simulink model "TosAeb"  (R2021a)
settings: Solver=FixedStepDiscrete  StartTime=0.0  StopTime=inf  FixedStep=0.01  RelTol=1e-3
undefined symbols (not defined in the file or in its folder): ACCModSts, AccSts1, AEB_ObjectMovingOrientation, AEB_ObjectMovingState, CN_BTCActive, CN_CurrentGearR, CN_CurrentGearR_DHT, CN_PTCActive, CN_VDCActive, CN_VSC_EspOff, CN_VSC_FCW_AEB_Error, CN_VSC_FCW_AEB_NoDispay, CN_VSC_FCW_AEB_PerfmDgdtn, CN_VSC_FCW_AEB_TempUnAvlbl, CN_VSC_VehStandstill, CNaccelAvoidMax, CNaccelAvoidMin, CNbrakePedalPress, CNcomfortStrLeftAccelMax, CNcomfortStrLeftAccelMin, CNcomfortStrRightAccelMax, CNcomfortStrRightAccelMin, CNDrvDoorOpen, CNDrvModeEco, CNDrvModeNorm, CNDrvModeOther, CNDrvModeSport, CNDrvSBRreminder, CNNull, CNobjInvalidID, CNPassDoorOpen, CNPassSBRreminder, CNPEBSAccelNegInf, CNPEBSAccelNull, CNPEBSTimeInf, CNPEBSTimeNull, CNSTOPMAX, CNSTOPNULL, CNsubjWidth, CNSwtOFF, CNSwtON, CNTTCMAX, CNTTCNULL, CNVehConfigAEBCycNone, CNVehConfigAEBNone, CNVehConfigAEBPedNone, CNVehConfigFCWNone, CNVehConfigJANone, CollisionSeverity, FALSE, FCWSnvty, JACycObjCalcInfoDefaultParams, JAPedObjCalcInfoDefaultParams, JAVehObjCalcInfoDefaultParams, latCycObjCalcInfoDefaultParams, latPedObjCalcInfoDefaultParams, longObjCalcInfoDefaultParams, ModSts, movingStatus, ObjectType ... (+1112)
libraries: simulink x3031, AEBSFunctionLib x50, Ta_Library x33, AEBSStateMachineLib x23, Mylibrary x12, simulink_extras x7, ObjSelect_lib x3, dspstat3 x2
(part 82 of 102)

== system TosAeb/AEB_Objsel/TA_Processor/AEB_ObjSelect/TargetInfoCheck/Data_precess/TA_EffectCalculate/Obj_FilterFlgCalculate/Long_ObjFilter/TTR_Calculate/CalculateTTRAndTTM_else/LongitudinalTTRComputer/SelectTTI/ObjectOnlyWillStop ==
  StationaryObjectTTI : Inport
  StationaryObjectSolutionExists : Inport  Port=2
  Action Port : ActionPort  ActionPortLabel=elseif(u3~=0)
  NoOperation : Subsystem  mask(noop){}
      mask help: No operation block to to hadle when a signal is passed unchanged between ports with different names
      mask Display: disp('NOOP')
  NoOperation1 : Subsystem  mask(noop){}
      mask help: No operation block to to hadle when a signal is passed unchanged between ports with different names
      mask Display: disp('NOOP')
  TimeToIntersection : Outport
  SolutionExists : Outport  Port=2
  wires:
  NoOperation/1(Out) -> TimeToIntersection/1  "TimeToIntersection"
  StationaryObjectTTI/1 -> NoOperation/1(In)
  NoOperation1/1(Out) -> SolutionExists/1  "SolutionExists"
  StationaryObjectSolutionExists/1 -> NoOperation1/1(In)

== system TosAeb/AEB_Objsel/TA_Processor/AEB_ObjSelect/TargetInfoCheck/Data_precess/TA_EffectCalculate/Obj_FilterFlgCalculate/Long_ObjFilter/TTR_Calculate/CalculateTTRAndTTM_else/Obj_AbsInfo/LongitudinalEdgeToEdgeDistances/Rename ==
  note: Block intended for fooling the model advisor, which want the signal names to
      | match the port names and which only allows signal renaming in combination
      | with transformative operations. The block has no effect on the signal and is
      | intended to replace, e.g., gain blocks with one as its multiplication factor.
      | See MAAB jm_0010: Port block names in Simulink models.
  Name_in : Inport
      settings: AttributesFormatString=DT=%<OutDataTypeStr>
  Name_out : Outport
      settings: AttributesFormatString=DT=%<OutDataTypeStr>
  wires:
  Name_in/1 -> Name_out/1  "Name"

== system TosAeb/AEB_Objsel/TA_Processor/AEB_ObjSelect/TargetInfoCheck/Data_precess/TA_EffectCalculate/Obj_FilterFlgCalculate/Long_ObjFilter/TTR_Calculate/CalculateTTRAndTTM_else/Obj_AbsInfo/LongitudinalEdgeToEdgeDistances/Rename1 ==
  note: Block intended for fooling the model advisor, which want the signal names to
      | match the port names and which only allows signal renaming in combination
      | with transformative operations. The block has no effect on the signal and is
      | intended to replace, e.g., gain blocks with one as its multiplication factor.
      | See MAAB jm_0010: Port block names in Simulink models.
  Name_in : Inport
      settings: AttributesFormatString=DT=%<OutDataTypeStr>
  Name_out : Outport
      settings: AttributesFormatString=DT=%<OutDataTypeStr>
  wires:
  Name_in/1 -> Name_out/1  "Name"

== system TosAeb/AEB_Objsel/TA_Processor/AEB_ObjSelect/TargetInfoCheck/Data_precess/TA_EffectCalculate/Obj_FilterFlgCalculate/Long_ObjFilter/TTR_Calculate/CalculateTTRAndTTM_else/Obj_AbsInfo/LongitudinalMotionData/Subsystem ==
  VehSelfData : Inport
  Add : Sum
  Add1 : Sum
  Atan2 : Trigonometry  Operator=atan2
  Bus Selector3 : BusSelector  OutputSignals=VehSelf.Veh_DriverAcc,VehSelf.YawRate,VehSelf.Veh_LongSpd_mps,VehSelf.Veh_AEBFunState
  Compare To Constant : simulink/Logic and Bit Operations/Compare To Constant  relop===  const=1  OutDataTypeStr=boolean
  Constant : Constant  Value=pi/2  OutDataTypeStr=single
  Constant16 : Constant  Value=P_TA_PrmForVehRearAxleDisttoFrnt
  MapToAngularInterval : Ta_Library/MapToAngularInterval  SystemSampleTime=-1  Latency=0  AutoFrameSizeCalculation=off
  Product : Product
  Subsystem1 : Subsystem
  Obj_AbsVx : Outport
  Subj_Vx : Outport  Port=2
  Obj_RefAcclx : Outport  Port=3
  Obj_AbsAcclx : Outport  Port=4
  Subj_Ax : Outport  Port=5
  wires:
  Product/1 -> Add1/2
  Compare To Constant/1 -> [AEB_Active]
  Subsystem1/2(Obj_AbsAcclx) -> Obj_AbsAcclx/1
  Bus Selector3/4 -> Compare To Constant/1  "<Veh_AEBFunState>"
  Bus Selector3/3 -> Subj_Vx/1, Add/2  "<Veh_LongSpd_mps>"
  Add/1 -> Obj_AbsVx/1, Atan2/2
  VehSelfData/1 -> Bus Selector3/1
  [Obj_Acc] -> Subsystem1/2(TA_ObjAccel)
  Constant/1 -> MapToAngularInterval/2
  [AEB_Active] -> Subsystem1/1(AEB)
  [Obj_vy] -> Add1/1
  Subsystem1/1(Obj_RefAcclx) -> Obj_RefAcclx/1
  MapToAngularInterval/1 -> Subsystem1/4(MappedAngle)
  Add1/1 -> Atan2/1
  [Obj_Vx] -> Add/1
  [Acc_x] -> Subsystem1/3(Subj_Ax)
  Atan2/1 -> MapToAngularInterval/1
  Bus Selector3/1 -> Subj_Ax/1, [Acc_x]  "<Veh_DriverAcc>"
  Bus Selector3/2 -> Product/1  "<YawRate>"
  Constant16/1 -> Product/2

== system TosAeb/AEB_Objsel/TA_Processor/AEB_ObjSelect/TargetInfoCheck/Data_precess/TA_EffectCalculate/Obj_FilterFlgCalculate/Long_ObjFilter/TTR_Calculate/CalculateTTRAndTTM_else/Obj_AbsInfo/StoppingDataForTTICalculation/LimitedSafeDivide ==
  note: 1e10
  note: 1e10
  Numerator : Inport
  Denominator : Inport  Port=2
  Constant1 : Constant  Value=1e10  OutDataTypeStr=single
  Constant5 : Constant  Value=1e10  OutDataTypeStr=single
  Gain : Gain  Gain=-1  OutDataTypeStr=Inherit: Same as input
  SafeDivide : Subsystem  TreatAsAtomicUnit=on  mask(Safe divide){DataType=single, DataTypeMax=0, DataTypeMin=0}
      mask prompts: DataType "Data type:"
      mask help:
      | This block offers division protected from division by zero.
      |
      | When division by zero is detected, a very large number will be returned with the same sign as the numerator.
      |
      | In the case zero divided by zero, the output has been defined as zero.
      |
      | Both floating-point numbers and integers are supported.
      mask init: same as TosAeb/AEB_Objsel/TA_Processor/PreProcessor/VehiclePreProcessor/CalculateCurvature/SafeDivide
      settings: RTWSystemCode=Inline
      mask Help:
      | This block offers division protected from division by zero.
      | 
      | When division by zero is detected, a very large number will be returned with the same sign as the numerator.
      | 
      | In the case zero divided by zero, the output has been defined as zero.
      | 
      | Both floating-point numbers and integers are supported.
      mask Display:
      | % Author: Jakob Andersson (jande573)
      | 
      | % Display the allowed data type
      | disp(DataType);
      | 
      | % Display the port symbols
      | port_label('input',1,'\bullet','texmode','on');
      | port_label('input',2,'\div','texmode','on');
  Saturation Dynamic : Subsystem  mask(Saturation Dynamic){OutMin=[], OutMax=[], OutDataTypeStr=Inherit: Same as second input, OutputDataTypeScalingMode=Same as second input, OutDataType=fixdt(1, 16), OutScaling=2^-10, LockScale=off, RndMeth=Floor, DoSatur=off}
      mask prompts: OutMin "Simulink:masks:OutputMinimum_MP"; OutMax "Simulink:masks:OutputMaximum_MP"; OutDataTypeStr "Simulink:masks:OutputDataType_MP"; OutputDataTypeScalingMode "Simulink:masks:OutputDataTypeMode_MP"; OutDataType "Simulink:masks:OutputDataTypeegFixdt116Fixdtsingle_MP"; OutScaling "Simulink:masks:OutputScalingSlopeEg29OrSlopeBias_MP"; LockScale "Simulink:masks:LockOutputDataTypeAgainstFxpTools_MP"; RndMeth "Simulink:masks:IntegerRoundingMode_MP"; DoSatur "Simulink:masks:SaturateOnIntegerOverflow_MP"
      mask help: Simulink:masks:SaturationDynamic_MD
      mask init: same as TosAeb/AEB_Objsel/TA_Processor/AEB_ObjSelect/TargetInfoCheck/Data_precess/TA_EffectCalculate/ValideateObj/ObjPos_Calculate/ObjPos_Calculateelse/InPath_Cal/DetermineInPath/LimitedSafeDivide/Saturation Dynamic
      mask Callback OutDataTypeStr: fixptdialog(gcbh)
      mask TypeOptions OutputDataTypeScalingMode: double | single | int8 | uint8 | int16 | uint16 | int32 | uint32 | Specify via dialog | Same as second input
      mask Callback OutputDataTypeScalingMode: fixptdialog(gcbh)
      mask Callback OutDataType: fixptdialog(gcbh)
      mask TypeOptions RndMeth: Simulink:masks:Ceiling | Simulink:masks:Convergent | Simulink:masks:Floor | Simulink:masks:Nearest | Simulink:masks:Round | Simulink:masks:Simplest | Simulink:masks:Zero
      mask Display: plot([0.15 0.85],[.5 .5],[.5 .5],[0.05 0.95],0.15+0.7*[0 .25 .75 1],0.05+0.9*[.25 .25 .75 .75]);
  LimitedQuotient : Outport
  wires:
  Gain/1 -> Saturation Dynamic/3(lo)
  Constant1/1 -> Gain/1
  Constant5/1 -> Saturation Dynamic/1(up)
  Saturation Dynamic/1(y) -> LimitedQuotient/1
  SafeDivide/1(Quotient) -> Saturation Dynamic/2(u)
  Denominator/1 -> SafeDivide/2(Denominator)
  Numerator/1 -> SafeDivide/1(Numerator)

== system TosAeb/AEB_Objsel/TA_Processor/AEB_ObjSelect/TargetInfoCheck/Data_precess/TA_EffectCalculate/Obj_FilterFlgCalculate/Long_ObjFilter/TTR_Calculate/CalculateTTRAndTTM_else/Obj_AbsInfo/StoppingDataForTTICalculation/LimitedSafeDivide1 ==
  note: 1e10
  note: 1e10
  Numerator : Inport
  Denominator : Inport  Port=2
  Constant1 : Constant  Value=1e10  OutDataTypeStr=single
  Constant5 : Constant  Value=1e10  OutDataTypeStr=single
  Gain : Gain  Gain=-1  OutDataTypeStr=Inherit: Same as input
  SafeDivide : Subsystem  TreatAsAtomicUnit=on  mask(Safe divide){DataType=single, DataTypeMax=0, DataTypeMin=0}
      mask prompts: DataType "Data type:"
      mask help:
      | This block offers division protected from division by zero.
      |
      | When division by zero is detected, a very large number will be returned with the same sign as the numerator.
      |
      | In the case zero divided by zero, the output has been defined as zero.
      |
      | Both floating-point numbers and integers are supported.
      mask init: same as TosAeb/AEB_Objsel/TA_Processor/PreProcessor/VehiclePreProcessor/CalculateCurvature/SafeDivide
      settings: RTWSystemCode=Inline
      mask Help:
      | This block offers division protected from division by zero.
      | 
      | When division by zero is detected, a very large number will be returned with the same sign as the numerator.
      | 
      | In the case zero divided by zero, the output has been defined as zero.
      | 
      | Both floating-point numbers and integers are supported.
      mask Display:
      | % Author: Jakob Andersson (jande573)
      | 
      | % Display the allowed data type
      | disp(DataType);
      | 
      | % Display the port symbols
      | port_label('input',1,'\bullet','texmode','on');
      | port_label('input',2,'\div','texmode','on');
  Saturation Dynamic : Subsystem  mask(Saturation Dynamic){OutMin=[], OutMax=[], OutDataTypeStr=Inherit: Same as second input, OutputDataTypeScalingMode=Same as second input, OutDataType=fixdt(1, 16), OutScaling=2^-10, LockScale=off, RndMeth=Floor, DoSatur=off}
      mask prompts: OutMin "Simulink:masks:OutputMinimum_MP"; OutMax "Simulink:masks:OutputMaximum_MP"; OutDataTypeStr "Simulink:masks:OutputDataType_MP"; OutputDataTypeScalingMode "Simulink:masks:OutputDataTypeMode_MP"; OutDataType "Simulink:masks:OutputDataTypeegFixdt116Fixdtsingle_MP"; OutScaling "Simulink:masks:OutputScalingSlopeEg29OrSlopeBias_MP"; LockScale "Simulink:masks:LockOutputDataTypeAgainstFxpTools_MP"; RndMeth "Simulink:masks:IntegerRoundingMode_MP"; DoSatur "Simulink:masks:SaturateOnIntegerOverflow_MP"
      mask help: Simulink:masks:SaturationDynamic_MD
      mask init: same as TosAeb/AEB_Objsel/TA_Processor/AEB_ObjSelect/TargetInfoCheck/Data_precess/TA_EffectCalculate/ValideateObj/ObjPos_Calculate/ObjPos_Calculateelse/InPath_Cal/DetermineInPath/LimitedSafeDivide/Saturation Dynamic
      mask Callback OutDataTypeStr: fixptdialog(gcbh)
      mask TypeOptions OutputDataTypeScalingMode: double | single | int8 | uint8 | int16 | uint16 | int32 | uint32 | Specify via dialog | Same as second input
      mask Callback OutputDataTypeScalingMode: fixptdialog(gcbh)
      mask Callback OutDataType: fixptdialog(gcbh)
      mask TypeOptions RndMeth: Simulink:masks:Ceiling | Simulink:masks:Convergent | Simulink:masks:Floor | Simulink:masks:Nearest | Simulink:masks:Round | Simulink:masks:Simplest | Simulink:masks:Zero
      mask Display: plot([0.15 0.85],[.5 .5],[.5 .5],[0.05 0.95],0.15+0.7*[0 .25 .75 1],0.05+0.9*[.25 .25 .75 .75]);
  LimitedQuotient : Outport
  wires:
  Gain/1 -> Saturation Dynamic/3(lo)
  Constant1/1 -> Gain/1
  Constant5/1 -> Saturation Dynamic/1(up)
  Saturation Dynamic/1(y) -> LimitedQuotient/1
  SafeDivide/1(Quotient) -> Saturation Dynamic/2(u)
  Denominator/1 -> SafeDivide/2(Denominator)
  Numerator/1 -> SafeDivide/1(Numerator)

== system TosAeb/AEB_Objsel/TA_Processor/AEB_ObjSelect/TargetInfoCheck/Data_precess/TA_EffectCalculate/Obj_FilterFlgCalculate/Long_ObjFilter/TTR_Calculate/CalculateTTRAndTTM_else/Obj_AbsInfo/StoppingDataForTTICalculation/LinearObjectPositionPredictor ==
  note: 计算自车在当前加速度下，由运动至静止的刹停距离
  note: Constant acceleration prediction:
      | pos_pred = pos + vel*time + acc*time^2/2
      | vel_pred = vel + acc*time
      | acc_pred = acc
  Posn : Inport  OutDataTypeStr=single
      settings: AttributesFormatString=DT=%<OutDataTypeStr>
  V : Inport  Port=2  OutDataTypeStr=single
      settings: AttributesFormatString=DT=%<OutDataTypeStr>
  A : Inport  Port=3  OutDataTypeStr=single
      settings: AttributesFormatString=DT=%<OutDataTypeStr>
  Ti : Inport  Port=4  OutDataTypeStr=single
      settings: AttributesFormatString=DT=%<OutDataTypeStr>
  Constant : Constant  Value=single(0.5)  OutDataTypeStr=single
      settings: AttributesFormatString=DT=%<OutDataTypeStr>
  Product1 : Product  RndMeth=Zero
  Product4 : Product  Inputs=**  RndMeth=Zero
  Product5 : Product  Inputs=***  RndMeth=Zero
  Sum6 : Sum  Inputs=+++
  PosnPred : Outport  OutDataTypeStr=single
      settings: AttributesFormatString=DT=%<OutDataTypeStr>
  wires:
  Sum6/1 -> PosnPred/1  "PosnPred"
  Product1/1 -> Product5/2  "TimeSquared"
  Product4/1 -> Sum6/2  "PositionChangeFromVelocity"
  A/1 -> Product5/1  "A"
  Ti/1 -> Product4/2, Product1/1, Product1/2  "Ti"
  V/1 -> Product4/1  "V"
  Posn/1 -> Sum6/1  "Posn"
  Constant/1 -> Product5/3  "Denominator"
  Product5/1 -> Sum6/3  "PositionChangeFromAcceleration"

== system TosAeb/AEB_Objsel/TA_Processor/AEB_ObjSelect/TargetInfoCheck/Data_precess/TA_EffectCalculate/Obj_FilterFlgCalculate/Long_ObjFilter/TTR_Calculate/CalculateTTRAndTTM_else/Obj_AbsInfo/StoppingDataForTTICalculation/LinearObjectPositionPredictor1 ==
  note: 计算目标在当前加速度下，由运动到静止的行驶距离
  note: Constant acceleration prediction:
      | pos_pred = pos + vel*time + acc*time^2/2
      | vel_pred = vel + acc*time
      | acc_pred = acc
  Posn : Inport  OutDataTypeStr=single
      settings: AttributesFormatString=DT=%<OutDataTypeStr>
  V : Inport  Port=2  OutDataTypeStr=single
      settings: AttributesFormatString=DT=%<OutDataTypeStr>
  A : Inport  Port=3  OutDataTypeStr=single
      settings: AttributesFormatString=DT=%<OutDataTypeStr>
  Ti : Inport  Port=4  OutDataTypeStr=single
      settings: AttributesFormatString=DT=%<OutDataTypeStr>
  Constant : Constant  Value=single(0.5)  OutDataTypeStr=single
      settings: AttributesFormatString=DT=%<OutDataTypeStr>
  Product1 : Product  RndMeth=Zero
  Product4 : Product  Inputs=**  RndMeth=Zero
  Product5 : Product  Inputs=***  RndMeth=Zero
  Sum6 : Sum  Inputs=+++
  PosnPred : Outport  OutDataTypeStr=single
      settings: AttributesFormatString=DT=%<OutDataTypeStr>
  wires:
  Sum6/1 -> PosnPred/1  "PosnPred"
  Product1/1 -> Product5/2  "TimeSquared"
  Product4/1 -> Sum6/2  "PositionChangeFromVelocity"
  A/1 -> Product5/1  "A"
  Ti/1 -> Product4/2, Product1/1, Product1/2  "Ti"
  V/1 -> Product4/1  "V"
  Posn/1 -> Sum6/1  "Posn"
  Constant/1 -> Product5/3  "Denominator"
  Product5/1 -> Sum6/3  "PositionChangeFromAcceleration"

== system TosAeb/AEB_Objsel/TA_Processor/AEB_ObjSelect/TargetInfoCheck/Data_precess/TA_EffectCalculate/ValideateObj/ObjPos_Calculate/ObjPos_Calculateelse/InPath_Cal/DetermineInPath/LimitedSafeDivide/SafeDivide ==
  Numerator : Inport  OutDataTypeStr=DataType
      settings: AttributesFormatString=DT=%<OutDataTypeStr>
  Denominator : Inport  Port=2  OutDataTypeStr=DataType
      settings: AttributesFormatString=DT=%<OutDataTypeStr>
  SafeDivide : MATLAB Function
  Quotient : Outport  OutDataTypeStr=DataType
      settings: AttributesFormatString=DT=%<OutDataTypeStr>
  wires:
  SafeDivide/1(Quotient) -> Quotient/1
  Denominator/1 -> SafeDivide/2(Denominator)  "Denominator"
  Numerator/1 -> SafeDivide/1(Numerator)  "Numerator"

== system TosAeb/AEB_Objsel/TA_Processor/AEB_ObjSelect/TargetInfoCheck/Data_precess/TA_EffectCalculate/ValideateObj/ObjPos_Calculate/ObjPos_Calculateelse/InPath_Cal/DetermineInPath/LimitedSafeDivide/Saturation Dynamic ==
  up : Inport
  u : Inport  Port=2
  lo : Inport  Port=3
  Data Type Duplicate : DataTypeDuplicate  DisableCoverage=on
  Data Type Propagation : S-Function  FunctionName=sfix_dtprop  Parameters=14,PropDataTypeMode,(getdatatypespecs(PropDataType,PropScaling,DblOver,0, 0, PropDataTypeMode, PropScalingMode)),IfRefDouble,IfRefSingle,IsSigned,NumBitsBase,NumBitsMult,NumBitsAdd,(sort(reshape(round(NumBitsAllowFinal), prod(size(NumBitsAllowFinal)),1))),PropScalingMode,ValuesUsedBestPrec,SlopeBase,SlopeMult,SlopeAdd,BiasBase,BiasMult,BiasAdd,DblOver  SFunctionDeploymentMode=off  EnableBusSupport=off  mask(Data Type Propagation){PropDataTypeMode=Inherit via propagation rule, PropDataType=fixdt(1, 16), IfRefDouble=double, IfRefSingle=single, IsSigned=IsSigned1, NumBitsBase=NumBits1, NumBitsMult=1, NumBitsAdd=0, NumBitsAllowFinal=1:128, PropScalingMode=Inherit via propagation rule, PropScaling=2^-10, ValuesUsedBestPrec=[5 -7], SlopeBase=Slope1, SlopeMult=1, SlopeAdd=0, BiasBase=Bias1, BiasMult=1, BiasAdd=0}
      mask prompts: PropDataTypeMode "Simulink:masks:x1PropagatedDataType_MP"; PropDataType "Simulink:masks:x11PropagatedDataTypeegFixdt116Fixdtsingle_MP"; IfRefDouble "Simulink:masks:x11IfAnyReferenceInputIsDoubleOutputIs_MP"; IfRefSingle "Simulink:masks:x12IfAnyReferenceInputIsSingleOutputIs_MP"; IsSigned "Simulink:masks:x13IsSigned_MP"; NumBitsBase "Simulink:masks:x141NumberofBitsBase_MP"; NumBitsMult "Simulink:masks:x142NumberofBitsMultiplicativeAdjustment_MP"; NumBitsAdd "Simulink:masks:x143NumberofBitsAdditiveAdjustment_MP"; NumBitsAllowFinal "Simulink:masks:x144NumberofBitsAllowableFinalValues_MP"; PropScalingMode "Simulink:masks:x2PropagatedScaling_MP"; PropScaling "Simulink:masks:x21PropagatedScalingSlopeEg29OrSlopeBiasEg1253_MP"; ValuesUsedBestPrec "Simulink:masks:x21ValuesUsedToDetermineBestPrecisionScaling_MP"; SlopeBase "Simulink:masks:x211SlopeBase_MP"; SlopeMult "Simulink:masks:x212SlopeMultiplicativeAdjustment_MP"; SlopeAdd "Simulink:masks:x213SlopeAdditiveAdjustment_MP"; BiasBase "Simulink:masks:x221BiasBase_MP"; BiasMult "Simulink:masks:x222BiasMultiplicativeAdjustment_MP"; BiasAdd "Simulink:masks:x223BiasAdditiveAdjustment_MP"
      mask help: Simulink:masks:DataTypePropagation_MD
      mask init:
      | DblOver = 0;
      mask TypeOptions PropDataTypeMode: Simulink:masks:SpecifyViaDialog | Simulink:masks:InheritViaPropagationRule
      mask Callback PropDataTypeMode: fixptdialog(gcbh)
      mask Callback PropDataType: fixptdialog(gcbh)
      mask TypeOptions IfRefDouble: Simulink:masks:double | Simulink:masks:single
      mask TypeOptions IfRefSingle: Simulink:masks:double | Simulink:masks:single
      mask TypeOptions IsSigned: Simulink:masks:IsSigned1 | Simulink:masks:IsSigned2 | Simulink:masks:IsSigned1OrIsSigned2 | Simulink:masks:TRUE | Simulink:masks:FALSE
      mask TypeOptions NumBitsBase: NumBits1 | NumBits2 | max([NumBits1 NumBits2]) | min([NumBits1 NumBits2]) | NumBits1+NumBits2
      mask TypeOptions PropScalingMode: Simulink:masks:SpecifyViaDialog | Simulink:masks:InheritViaPropagationRule | Simulink:masks:ObtainViaBestPrecision
      mask Callback PropScalingMode: fixptdialog(gcbh)
      mask TypeOptions SlopeBase: Slope1 | Slope2 | max([Slope1 Slope2]) | min([Slope1 Slope2]) | Slope1*Slope2 | Slope1/Slope2 | PosRange1 | PosRange2 | max([PosRange1 PosRange2]) | min([PosRange1 PosRange2]) | PosRange1*PosRange2 | PosRange1/PosRange2
      mask TypeOptions BiasBase: Bias1 | Bias2 | max([Bias1 Bias2]) | min([Bias1 Bias2]) | Bias1*Bias2 | Bias1/Bias2 | Bias1+Bias2 | Bias1-Bias2
      mask Display:
      | port_label('Input',1,'Ref1');
      | port_label('Input',2,'Ref2');
      | port_label('Input',3,'Prop');
  LowerRelop1 : RelationalOperator  DisableCoverage=on  Operator=>  OutDataTypeStr=boolean  RndMeth=Nearest
  Switch : Switch  Criteria=u2 ~= 0  OutDataTypeStr=Inherit: Inherit via back propagation  ZeroCross=off
  Switch2 : Switch  Criteria=u2 ~= 0  OutMin=OutMin  OutMax=OutMax  OutDataTypeStr=Inherit: Inherit via back propagation  ZeroCross=off
  UpperRelop : RelationalOperator  DisableCoverage=on  Operator=<  OutDataTypeStr=boolean  RndMeth=Nearest
  y : Outport  OutMin=OutMin  OutMax=OutMax
  wires:
  up/1 -> Switch2/1, LowerRelop1/2
  u/1 -> Data Type Propagation/2, Data Type Propagation/1, LowerRelop1/1, Switch/3, UpperRelop/1
  lo/1 -> Switch/1, UpperRelop/2
  Switch/1 -> Data Type Duplicate/2, Switch2/3, Data Type Propagation/3
  Switch2/1 -> Data Type Duplicate/1, y/1
  LowerRelop1/1 -> Switch2/2
  UpperRelop/1 -> Switch/2

== system TosAeb/AEB_Objsel/TA_Processor/AEB_ObjSelect/TargetInfoCheck/Data_precess/TA_EffectCalculate/ValideateObj/ObjPos_Calculate/ObjPos_Calculateelse/InPath_Cal/DetermineInPath/PredictHostLateralPositionAtTTR/CalculateHeading ==
  note: If the current point is in segment i, and the speed is close to zero,
      | then the speed at the start point of segment i, i-1,...,1 are checked for non-zero speed.
      | The first non-zero speed found is used as basis for calculating the heading angle.
      | If all checked points have zero speed, then the heading is set to zero.
  PathEstimn : Inport  OutDataTypeStr=Bus: PahEstimn
  SegmentIndex : Inport  Port=2
  dx : Inport  Port=3
  dy : Inport  Port=4
  CheckSpeed : Subsystem
  ExtractVelocitiesAtStartOfSegments : Subsystem  TreatAsAtomicUnit=on
  FindValidIndex : MATLAB Function  TreatAsAtomicUnit=on
  FindValidVelocityVector : MATLAB Function  TreatAsAtomicUnit=on
  atan2 : Trigonometry  Operator=atan2
  PredHostHeading : Outport
  wires:
  ExtractVelocitiesAtStartOfSegments/3(SegmentVelocitiesY) -> FindValidVelocityVector/6(SegmentVelocitiesY)
  ExtractVelocitiesAtStartOfSegments/2(SegmentVelocitiesX) -> FindValidVelocityVector/5(SegmentVelocitiesX)
  ExtractVelocitiesAtStartOfSegments/1(SpeedValid) -> FindValidIndex/2(SpeedValid)
  FindValidVelocityVector/1(ValidVelocityY) -> atan2/1
  FindValidVelocityVector/2(ValidVelocityX) -> atan2/2
  CheckSpeed/1(SpeedValid) -> FindValidVelocityVector/1(CurrentVelocityValid)
  FindValidIndex/1(ValidSegmentIndex) -> FindValidVelocityVector/2(ValidSegmentIndex)
  [dx] -> FindValidVelocityVector/3(CurrentVelocityX)
  [dy] -> FindValidVelocityVector/4(CurrentVelocityY)
  SegmentIndex/1 -> FindValidIndex/1(SegmentIndex)
  atan2/1 -> PredHostHeading/1  "PredHostHeading"
  PathEstimn/1 -> ExtractVelocitiesAtStartOfSegments/1(PathEstimn)
  dx/1 -> CheckSpeed/1(x), [dx]
  dy/1 -> CheckSpeed/2(y), [dy]

== system TosAeb/AEB_Objsel/TA_Processor/AEB_ObjSelect/TargetInfoCheck/Data_precess/TA_EffectCalculate/ValideateObj/ObjPos_Calculate/ObjPos_Calculateelse/InPath_Cal/DetermineInPath/PredictHostLateralPositionAtTTR/SegmentComponents ==
  note: If the time is large, then it is possible that time exceeds "time end".
      | In that case, we assume time is time end
  Segment : Inport
  PredictionTime : Inport  Port=2
  SegmentIndex : Inport  Port=3
  Add1 : Sum  Inputs=+-
  Bus Selector : BusSelector  OutputSignals=CtrlAryLgt,CtrlAryLat,TiAry
  SafeDivide : Subsystem  TreatAsAtomicUnit=on  mask(Safe divide){DataType=single, DataTypeMax=0, DataTypeMin=0}
      mask prompts: DataType "Data type:"
      mask help:
      | This block offers division protected from division by zero.
      |
      | When division by zero is detected, a very large number will be returned with the same sign as the numerator.
      |
      | In the case zero divided by zero, the output has been defined as zero.
      |
      | Both floating-point numbers and integers are supported.
      mask init: same as TosAeb/AEB_Objsel/TA_Processor/PreProcessor/VehiclePreProcessor/CalculateCurvature/SafeDivide
      settings: RTWSystemCode=Inline
      mask Help:
      | This block offers division protected from division by zero.
      | 
      | When division by zero is detected, a very large number will be returned with the same sign as the numerator.
      | 
      | In the case zero divided by zero, the output has been defined as zero.
      | 
      | Both floating-point numbers and integers are supported.
      mask Display:
      | % Author: Jakob Andersson (jande573)
      | 
      | % Display the allowed data type
      | disp(DataType);
      | 
      | % Display the port symbols
      | port_label('input',1,'\bullet','texmode','on');
      | port_label('input',2,'\div','texmode','on');
  Saturation : Saturate  UpperLimit=1  LowerLimit=0  OutDataTypeStr=single
      settings: AttributesFormatString=LowerLimit=%<LowerLimit>\nUpperLimit=%<UpperLimit>
  Saturation1 : Saturate  UpperLimit=inf  LowerLimit=single(0.001)  OutDataTypeStr=single
      settings: AttributesFormatString=LowerLimit=%<LowerLimit>\nUpperLimit=%<UpperLimit>
  Selector : Selector  InputPortWidth=4  IndexOptions=Index vector (dialog)  Indices=1  OutputSizes=1
  Selector1 : Selector  InputPortWidth=4  IndexOptions=Index vector (dialog)  Indices=2  OutputSizes=1
  Selector10 : Selector  InputPortWidth=4  IndexOptions=Index vector (port)  Indices=[1 3]  OutputSizes=1
  Selector2 : Selector  InputPortWidth=4  IndexOptions=Index vector (dialog)  Indices=3  OutputSizes=1
  Selector3 : Selector  InputPortWidth=4  IndexOptions=Index vector (dialog)  Indices=4  OutputSizes=1
  Selector4 : Selector  InputPortWidth=4  IndexOptions=Index vector (dialog)  Indices=1  OutputSizes=1
  Selector5 : Selector  InputPortWidth=4  IndexOptions=Index vector (dialog)  Indices=2  OutputSizes=1
  Selector6 : Selector  InputPortWidth=4  IndexOptions=Index vector (dialog)  Indices=3  OutputSizes=1
  Selector7 : Selector  InputPortWidth=4  IndexOptions=Index vector (dialog)  Indices=4  OutputSizes=1
  Selector8 : Selector  InputPortWidth=2  IndexOptions=Index vector (dialog)  Indices=1  OutputSizes=1
  Selector9 : Selector  InputPortWidth=2  IndexOptions=Index vector (dialog)  Indices=2  OutputSizes=1
  time duration : Sum  Inputs=-+
  P0x : Outport
  P1x : Outport  Port=2
  P2x : Outport  Port=3
  P3x : Outport  Port=4
  P0y : Outport  Port=5
  P1y : Outport  Port=6
  P2y : Outport  Port=7
  P3y : Outport  Port=8
  tau : Outport  Port=9
  wires:
  Saturation/1 -> tau/1  "tau"
  SafeDivide/1(Quotient) -> Saturation/1  "RelativeTime"
  time duration/1 -> Saturation1/1
  Add1/1 -> SafeDivide/1(Numerator)
  PredictionTime/1 -> Add1/1
  Selector8/1 -> Add1/2, time duration/1  "TimeStart"
  Selector9/1 -> time duration/2  "TimeEnd"
  Selector7/1 -> P3y/1  "P3y"
  Selector6/1 -> P2y/1  "P2y"
  Selector5/1 -> P1y/1  "P1y"
  Selector4/1 -> P0y/1  "P0y"
  Selector3/1 -> P3x/1  "P3x"
  Selector2/1 -> P2x/1  "P2x"
  Selector1/1 -> P1x/1  "P1x"
  Selector/1 -> P0x/1  "P0x"
  Saturation1/1 -> SafeDivide/2(Denominator)
  Segment/1 -> Selector10/1
  Bus Selector/1 -> Selector3/1, Selector2/1, Selector1/1, Selector/1  "<CtrlAryLgt>"
  Bus Selector/2 -> Selector5/1, Selector6/1, Selector7/1, Selector4/1  "<CtrlAryLat>"
  Bus Selector/3 -> Selector9/1, Selector8/1  "<TiAry>"
  SegmentIndex/1 -> Selector10/2
  Selector10/1 -> Bus Selector/1

== system TosAeb/AEB_Objsel/TA_Processor/AEB_ObjSelect/TargetInfoCheck/Data_precess/TA_EffectCalculate/ValideateObj/ObjPos_Calculate/ObjPos_Calculateelse/InPath_Cal/DetermineInPath/PredictHostLateralPositionAtTTR/SelectSegment ==
  note: The purpose is to choose that segment such that:
      | Path.T1 < Time <= Path.T2
      | However if Time is large, i.e. Time > Path.T2
      | for all Path.Segments then choose last Path
  note: Maximum one element can be true
      | in the TimeWithinSegment vector.
  PahEstimn : Inport
  Time : Inport  Port=2
  Constant : Constant  Value=4  OutDataTypeStr=uint8
  ForEachSegmentInPahEstimn : Subsystem  TreatAsAtomicUnit=on
  Indices : Constant  Value=uint8([1:4])
  Logop_1 : Logic  Operator=OR  Inputs=1  AllPortsSameDT=off  OutDataTypeStr=boolean
  Max of Elements : MinMax  Function=max
  Product : Product  RndMeth=Zero
  Sum of Elements2 : Sum  Commented=on  Inputs=+
  Switch : Switch  Criteria=u2 ~= 0
  SegmentIndex : Outport
  wires:
  PahEstimn/1 -> ForEachSegmentInPahEstimn/1(PahEstimn)
  Time/1 -> ForEachSegmentInPahEstimn/2(Time)
  Switch/1 -> SegmentIndex/1
  ForEachSegmentInPahEstimn/1(TimeWithinSegment) -> Logop_1/1, Product/2
  Logop_1/1 -> Switch/2  "OneSegmentFound"
  Indices/1 -> Product/1
  Product/1 -> Max of Elements/1
  Max of Elements/1 -> Switch/1
  Constant/1 -> Switch/3

== system TosAeb/AEB_Objsel/TA_Processor/AEB_ObjSelect/TargetInfoCheck/Data_precess/TA_EffectCalculate/ValideateObj/ObjPos_Calculate/ObjPos_Calculateelse/InPath_Cal/DetermineInPath/PredictObjectLateralPositionAtTTR/Rename ==
  note: Block intended for fooling the model advisor, which want the signal names to
      | match the port names and which only allows signal renaming in combination
      | with transformative operations. The block has no effect on the signal and is
      | intended to replace, e.g., gain blocks with one as its multiplication factor.
      | See MAAB jm_0010: Port block names in Simulink models.
  Name_in : Inport
      settings: AttributesFormatString=DT=%<OutDataTypeStr>
  Name_out : Outport
      settings: AttributesFormatString=DT=%<OutDataTypeStr>
  wires:
  Name_in/1 -> Name_out/1  "Name"

== system TosAeb/AEB_Objsel/TA_Processor/PreProcessor/ObjectPrecessor/TAObjInfoCalc/For Each Subsystem/Info_Check1/BasicInfo_check/DxCorrect/dx_charge/Dx_Flag/dx_compare/DistancebyDx ==
  Fus_ObjDx_Single : Inport
  Add : Sum  Inputs=+-
  Delay : Delay  DelayLength=5
  DisbyDx : Outport
  wires:
  Add/1 -> DisbyDx/1
  Fus_ObjDx_Single/1 -> Delay/1, Add/1
  Delay/1 -> Add/2

== system TosAeb/AEB_Objsel/TA_Processor/PreProcessor/ObjectPrecessor/TAObjInfoCalc/For Each Subsystem/Info_Check1/BasicInfo_check/DxCorrect/dx_charge/Dx_Flag/dx_compare/DistancebyVx ==
  Fus_ObjVx_Single : Inport
  Constant : Constant  Value=5  OutDataTypeStr=single
  Constant1 : Constant  Value=0.25  OutDataTypeStr=single
  Divide : Product  Inputs=*/
  Product : Product
  Sum of Elements : Sum  Inputs=+
  Tapped Delay : simulink/Discrete/Tapped Delay  vinit=0.0  samptime=-1  NumDelays=4  DelayOrder=Oldest  includeCurrent=off
  DisbyVx : Outport
  wires:
  Constant/1 -> Divide/2
  Fus_ObjVx_Single/1 -> Tapped Delay/1
  Divide/1 -> Product/1
  Sum of Elements/1 -> Divide/1
  Constant1/1 -> Product/2
  Tapped Delay/1 -> Sum of Elements/1
  Product/1 -> DisbyVx/1

== system TosAeb/AEB_Objsel/TA_Processor/PreProcessor/ObjectPrecessor/TAObjInfoCalc/For Each Subsystem/Info_Check1/BasicInfo_check/DxCorrect/dx_charge/Dx_Flag/dx_compare/distance_charge ==
  DisbyDx : Inport
  DisbyVx : Inport  Port=2
  Add : Sum
  Add1 : Sum  Inputs=+-
  Constant : Constant  Value=2
  Constant1 : Constant  Value=P_TA_ObjDistancebyVxandDxFactor
  Divide : Product  Inputs=*/
  Product : Product
  Relational Operator : RelationalOperator  OutDataTypeStr=boolean  RndMeth=Simplest
  DisLong_flg : Outport
  wires:
  DisbyDx/1 -> Add/1, Add1/1, Product/2
  DisbyVx/1 -> Add/2
  Add/1 -> Divide/1
  Constant/1 -> Divide/2
  Divide/1 -> Add1/2
  Constant1/1 -> Product/1
  Product/1 -> Relational Operator/1
  Add1/1 -> Relational Operator/2
  Relational Operator/1 -> DisLong_flg/1

== system TosAeb/AEB_Objsel/TA_Processor/PreProcessor/ObjectPrecessor/TAObjInfoCalc/For Each Subsystem/Info_Check1/BasicInfo_check/DyCorrect/dy_charge/Subsystem2/charge/DistancebyDy ==
  Fus_ObjDyQua_Single : Inport
  Add : Sum  Inputs=+-
  Delay : Delay  DelayLength=5
  DisbyDy : Outport
  wires:
  Delay/1 -> Add/2
  Fus_ObjDyQua_Single/1 -> Add/1, Delay/1
  Add/1 -> DisbyDy/1

== system TosAeb/AEB_Objsel/TA_Processor/PreProcessor/ObjectPrecessor/TAObjInfoCalc/For Each Subsystem/Info_Check1/BasicInfo_check/DyCorrect/dy_charge/Subsystem2/charge/DistancebyVy ==
  Fus_ObjVy_Single : Inport
  Constant : Constant  Value=5  OutDataTypeStr=single
  Constant1 : Constant  Value=0.25  OutDataTypeStr=single
  Divide : Product  Inputs=*/
  Product : Product
  Sum of Elements : Sum  Inputs=+
  Tapped Delay : simulink/Discrete/Tapped Delay  vinit=0.0  samptime=-1  NumDelays=4  DelayOrder=Oldest  includeCurrent=off
  DisbyVy : Outport
  wires:
  Product/1 -> DisbyVy/1
  Tapped Delay/1 -> Sum of Elements/1
  Constant1/1 -> Product/2
  Sum of Elements/1 -> Divide/1
  Divide/1 -> Product/1
  Fus_ObjVy_Single/1 -> Tapped Delay/1
  Constant/1 -> Divide/2

== system TosAeb/AEB_Objsel/TA_Processor/PreProcessor/ObjectPrecessor/TAObjInfoCalc/For Each Subsystem/Info_Check1/BasicInfo_check/DyCorrect/dy_charge/Subsystem2/charge/Subsystem ==
  DisbyDy : Inport
  DisbyVy : Inport  Port=2
  Add : Sum
  Add1 : Sum  Inputs=+-
  Constant : Constant  Value=2
  Constant1 : Constant  Value=P_TA_ObjDistancebyVyandDyFactor
  Divide : Product  Inputs=*/
  Product : Product
  Relational Operator : RelationalOperator  OutDataTypeStr=boolean  RndMeth=Simplest
  DisLat_flg : Outport
  wires:
  Relational Operator/1 -> DisLat_flg/1
  Add1/1 -> Relational Operator/2
  Product/1 -> Relational Operator/1
  Constant1/1 -> Product/1
  Divide/1 -> Add1/2
  Constant/1 -> Divide/2
  Add/1 -> Divide/1
  DisbyVy/1 -> Add/2
  DisbyDy/1 -> Product/2, Add1/1, Add/1

== system TosAeb/AEB_Objsel/TA_Processor/PreProcessor/ObjectPrecessor/TAObjInfoCalc/For Each Subsystem/Info_Check1/BasicInfo_check/VxCorrect/Subsystem3/Subsystem2/Subsystem/Subsystem ==
  VbyDx : Inport
  VbyVx : Inport  Port=2
  Add : Sum
  Add1 : Sum  Inputs=+-
  Constant : Constant  Value=2
  Constant1 : Constant  Value=P_TA_ObjSpeedxbyVxandDxFactor
  Divide : Product  Inputs=*/
  Divide1 : Product  Inputs=**
  Relational Operator : RelationalOperator  OutDataTypeStr=boolean  RndMeth=Simplest
  DisLong_flg : Outport
  wires:
  Divide1/1 -> Relational Operator/2
  Constant1/1 -> Divide1/2
  Relational Operator/1 -> DisLong_flg/1
  Add1/1 -> Relational Operator/1
  VbyDx/1 -> Add1/1, Add/1
  Divide/1 -> Add1/2
  Constant/1 -> Divide/2
  Add/1 -> Divide/1
  VbyVx/1 -> Divide1/1, Add/2

== system TosAeb/AEB_Objsel/TA_Processor/PreProcessor/ObjectPrecessor/TAObjInfoCalc/For Each Subsystem/Info_Check1/BasicInfo_check/VxCorrect/Subsystem3/Subsystem2/Subsystem/VbyDx ==
  Fus_ObjDx_Single : Inport
  Add : Sum  Inputs=+-
  Constant : Constant  Value=0.25  OutDataTypeStr=single
  Delay : Delay  DelayLength=5
  Divide : Product  Inputs=*/
  VbyDx : Outport
  wires:
  Constant/1 -> Divide/2
  Divide/1 -> VbyDx/1
  Add/1 -> Divide/1
  Delay/1 -> Add/2
  Fus_ObjDx_Single/1 -> Delay/1, Add/1

== system TosAeb/AEB_Objsel/TA_Processor/PreProcessor/ObjectPrecessor/TAObjInfoCalc/For Each Subsystem/Info_Check1/BasicInfo_check/VxCorrect/Subsystem3/Subsystem2/Subsystem/VbyVx ==
  Fus_ObjVx_Single : Inport
  Constant : Constant  Value=5  OutDataTypeStr=single
  Divide : Product  Inputs=*/
  Sum of Elements : Sum  Inputs=+
  Tapped Delay : simulink/Discrete/Tapped Delay  vinit=0.0  samptime=-1  NumDelays=4  DelayOrder=Oldest  includeCurrent=off
  VbyVx : Outport
  wires:
  Divide/1 -> VbyVx/1
  Tapped Delay/1 -> Sum of Elements/1
  Sum of Elements/1 -> Divide/1
  Fus_ObjVx_Single/1 -> Tapped Delay/1
  Constant/1 -> Divide/2

== system TosAeb/AEB_Objsel/TA_Processor/PreProcessor/ObjectPrecessor/TAObjInfoCalc/For Each Subsystem/Info_Check1/BasicInfo_check/VyCorrect/Subsystem3/Subsystem2/Subsystem/Subsystem ==
  VbyDy : Inport
  VbyVy : Inport  Port=2
  Add : Sum
  Add1 : Sum  Inputs=+-
  Constant : Constant  Value=2
  Constant1 : Constant  Value=P_TA_ObjSpeedybyVyandDyFactor
  Constant2 : Constant  Value=0  OutDataTypeStr=boolean
  Divide : Product  Inputs=*/
  Divide1 : Product  Inputs=**
  Relational Operator : RelationalOperator  OutDataTypeStr=boolean  RndMeth=Simplest
  Terminator : Terminator
  DisLat_flg : Outport
  wires:
  Constant2/1 -> DisLat_flg/1
  VbyVy/1 -> Add/2, Divide1/1
  Add/1 -> Divide/1
  Constant/1 -> Divide/2
  Divide/1 -> Add1/2
  VbyDy/1 -> Add/1, Add1/1
  Add1/1 -> Relational Operator/1
  Relational Operator/1 -> Terminator/1
  Constant1/1 -> Divide1/2
  Divide1/1 -> Relational Operator/2

== system TosAeb/AEB_Objsel/TA_Processor/PreProcessor/ObjectPrecessor/TAObjInfoCalc/For Each Subsystem/Info_Check1/BasicInfo_check/VyCorrect/Subsystem3/Subsystem2/Subsystem/VbyDy ==
  Fus_ObjDy_Single : Inport
  Add : Sum  Inputs=+-
  Constant : Constant  Value=0.25  OutDataTypeStr=single
  Delay : Delay  DelayLength=250
  Divide : Product  Inputs=*/
  VbyDy : Outport
  wires:
  Fus_ObjDy_Single/1 -> Add/1, Delay/1
  Delay/1 -> Add/2
  Add/1 -> Divide/1
  Divide/1 -> VbyDy/1
  Constant/1 -> Divide/2

== system TosAeb/AEB_Objsel/TA_Processor/PreProcessor/ObjectPrecessor/TAObjInfoCalc/For Each Subsystem/Info_Check1/BasicInfo_check/VyCorrect/Subsystem3/Subsystem2/Subsystem/VbyVy ==
  Fus_ObjVy_Single : Inport
  Constant : Constant  Value=5  OutDataTypeStr=single
  Divide : Product  Inputs=*/
  Sum of Elements : Sum  Inputs=+
  Tapped Delay : simulink/Discrete/Tapped Delay  vinit=0.0  samptime=-1  NumDelays=4  DelayOrder=Oldest  includeCurrent=off
  VbyVy : Outport
  wires:
  Constant/1 -> Divide/2
  Fus_ObjVy_Single/1 -> Tapped Delay/1
  Sum of Elements/1 -> Divide/1
  Tapped Delay/1 -> Sum of Elements/1
  Divide/1 -> VbyVy/1

== system TosAeb/AEB_Objsel/TA_Processor/PreProcessor/ObjectPrecessor/TAObjInfoCalc/For Each Subsystem/Info_Check1/Basic_Info_check/DxCorrect/dx_charge/Dx_Flag/dx_compare/DistancebyDx ==
  Fus_ObjDx_Single : Inport
  Add : Sum  Inputs=+-
  Delay : Delay  DelayLength=5
  DisbyDx : Outport
  wires:
  Add/1 -> DisbyDx/1
  Fus_ObjDx_Single/1 -> Delay/1, Add/1
  Delay/1 -> Add/2

== system TosAeb/AEB_Objsel/TA_Processor/PreProcessor/ObjectPrecessor/TAObjInfoCalc/For Each Subsystem/Info_Check1/Basic_Info_check/DxCorrect/dx_charge/Dx_Flag/dx_compare/DistancebyVx ==
  Fus_ObjVx_Single : Inport
  Constant : Constant  Value=5  OutDataTypeStr=single
  Constant1 : Constant  Value=0.25  OutDataTypeStr=single
  Delay : Delay
  Delay1 : Delay  DelayLength=3
  Delay2 : Delay  DelayLength=4
  Delay3 : Delay  DelayLength=5
  Divide : Product  Inputs=*/
  Product : Product
  Sum of Elements : Sum  Inputs=+++++
  Unit Delay : UnitDelay  SampleTime=-1  HasFrameUpgradeWarning=on
  DisbyVx : Outport
  wires:
  Constant/1 -> Divide/2
  Divide/1 -> Product/1
  Sum of Elements/1 -> Divide/1
  Constant1/1 -> Product/2
  Product/1 -> DisbyVx/1
  Unit Delay/1 -> Sum of Elements/1
  Delay/1 -> Sum of Elements/2
  Delay1/1 -> Sum of Elements/3
  Delay2/1 -> Sum of Elements/4
  Delay3/1 -> Sum of Elements/5
  Fus_ObjVx_Single/1 -> Delay3/1, Delay2/1, Delay1/1, Delay/1, Unit Delay/1

== system TosAeb/AEB_Objsel/TA_Processor/PreProcessor/ObjectPrecessor/TAObjInfoCalc/For Each Subsystem/Info_Check1/Basic_Info_check/DxCorrect/dx_charge/Dx_Flag/dx_compare/distance_charge ==
  DisbyDx : Inport
  DisbyVx : Inport  Port=2
  Add : Sum
  Add1 : Sum  Inputs=+-
  Constant : Constant  Value=2
  Constant1 : Constant  Value=P_TA_ObjDistancebyVxandDxFactor
  Divide : Product  Inputs=*/
  Product : Product
  Relational Operator : RelationalOperator  OutDataTypeStr=boolean  RndMeth=Simplest
  DisLong_flg : Outport
  wires:
  DisbyDx/1 -> Add/1, Add1/1, Product/2
  DisbyVx/1 -> Add/2
  Add/1 -> Divide/1
  Constant/1 -> Divide/2
  Divide/1 -> Add1/2
  Constant1/1 -> Product/1
  Product/1 -> Relational Operator/1
  Add1/1 -> Relational Operator/2
  Relational Operator/1 -> DisLong_flg/1

== system TosAeb/AEB_Objsel/TA_Processor/PreProcessor/ObjectPrecessor/TAObjInfoCalc/For Each Subsystem/Info_Check1/Basic_Info_check/DxCorrect1/dx_charge/Dx_Flag/dx_compare/DistancebyDx ==
  Fus_ObjDx_Single : Inport
  Add : Sum  Inputs=+-
  Delay : Delay  DelayLength=5
  DisbyDx : Outport
  wires:
  Delay/1 -> Add/2
  Fus_ObjDx_Single/1 -> Add/1, Delay/1
  Add/1 -> DisbyDx/1

== system TosAeb/AEB_Objsel/TA_Processor/PreProcessor/ObjectPrecessor/TAObjInfoCalc/For Each Subsystem/Info_Check1/Basic_Info_check/DxCorrect1/dx_charge/Dx_Flag/dx_compare/DistancebyVx ==
  Fus_ObjVx_Single : Inport
  Constant : Constant  Value=5  OutDataTypeStr=single
  Constant1 : Constant  Value=0.25  OutDataTypeStr=single
  Delay : Delay
  Delay1 : Delay  DelayLength=3
  Delay2 : Delay  DelayLength=4
  Delay3 : Delay  DelayLength=5
  Divide : Product  Inputs=*/
  Product : Product
  Sum of Elements : Sum  Inputs=+++++
  Unit Delay : UnitDelay  SampleTime=-1  HasFrameUpgradeWarning=on
  DisbyVx : Outport
  wires:
  Fus_ObjVx_Single/1 -> Unit Delay/1, Delay/1, Delay1/1, Delay2/1, Delay3/1
  Delay3/1 -> Sum of Elements/5
  Delay2/1 -> Sum of Elements/4
  Delay1/1 -> Sum of Elements/3
  Delay/1 -> Sum of Elements/2
  Unit Delay/1 -> Sum of Elements/1
  Product/1 -> DisbyVx/1
  Constant1/1 -> Product/2
  Sum of Elements/1 -> Divide/1
  Divide/1 -> Product/1
  Constant/1 -> Divide/2

== system TosAeb/AEB_Objsel/TA_Processor/PreProcessor/ObjectPrecessor/TAObjInfoCalc/For Each Subsystem/Info_Check1/Basic_Info_check/DxCorrect1/dx_charge/Dx_Flag/dx_compare/distance_charge ==
  DisbyDx : Inport
  DisbyVx : Inport  Port=2
  Add : Sum
  Add1 : Sum  Inputs=+-
  Constant : Constant  Value=2
  Constant1 : Constant  Value=P_TA_ObjDistancebyVxandDxFactor
  Divide : Product  Inputs=*/
  Product : Product
  Relational Operator : RelationalOperator  OutDataTypeStr=boolean  RndMeth=Simplest
  DisLong_flg : Outport
  wires:
  Relational Operator/1 -> DisLong_flg/1
  Add1/1 -> Relational Operator/2
  Product/1 -> Relational Operator/1
  Constant1/1 -> Product/1
  Divide/1 -> Add1/2
  Constant/1 -> Divide/2
  Add/1 -> Divide/1
  DisbyVx/1 -> Add/2
  DisbyDx/1 -> Product/2, Add1/1, Add/1

== system TosAeb/AEB_Objsel/TA_Processor/PreProcessor/ObjectPrecessor/TAObjInfoCalc/For Each Subsystem/Info_Check1/Basic_Info_check/DyCorrect/dy_charge/Subsystem2/charge/DistancebyDy ==
  Fus_ObjDyQua_Single : Inport
  Add : Sum  Inputs=+-
  Delay : Delay  DelayLength=5
  DisbyDy : Outport
  wires:
  Fus_ObjDyQua_Single/1 -> Delay/1, Add/1
  Add/1 -> DisbyDy/1
  Delay/1 -> Add/2

== system TosAeb/AEB_Objsel/TA_Processor/PreProcessor/ObjectPrecessor/TAObjInfoCalc/For Each Subsystem/Info_Check1/Basic_Info_check/DyCorrect/dy_charge/Subsystem2/charge/DistancebyVy ==
  Fus_ObjVy_Single : Inport
  Constant : Constant  Value=5  OutDataTypeStr=single
  Constant1 : Constant  Value=0.25  OutDataTypeStr=single
  Divide : Product  Inputs=*/
  Product : Product
  Sum of Elements : Sum  Inputs=+
  Tapped Delay : simulink/Discrete/Tapped Delay  vinit=0.0  samptime=-1  NumDelays=4  DelayOrder=Oldest  includeCurrent=off
  DisbyVy : Outport
  wires:
  Product/1 -> DisbyVy/1
  Tapped Delay/1 -> Sum of Elements/1
  Constant1/1 -> Product/2
  Sum of Elements/1 -> Divide/1
  Divide/1 -> Product/1
  Fus_ObjVy_Single/1 -> Tapped Delay/1
  Constant/1 -> Divide/2

== system TosAeb/AEB_Objsel/TA_Processor/PreProcessor/ObjectPrecessor/TAObjInfoCalc/For Each Subsystem/Info_Check1/Basic_Info_check/DyCorrect/dy_charge/Subsystem2/charge/Subsystem ==
  DisbyDy : Inport
  DisbyVy : Inport  Port=2
  Add : Sum
  Add1 : Sum  Inputs=+-
  Constant : Constant  Value=2
  Constant1 : Constant  Value=P_TA_ObjDistancebyVyandDyFactor
  Divide : Product  Inputs=*/
  Product : Product
  Relational Operator : RelationalOperator  OutDataTypeStr=boolean  RndMeth=Simplest
  DisLat_flg : Outport
  wires:
  Relational Operator/1 -> DisLat_flg/1
  Add1/1 -> Relational Operator/2
  Product/1 -> Relational Operator/1
  Constant1/1 -> Product/1
  Divide/1 -> Add1/2
  Constant/1 -> Divide/2
  Add/1 -> Divide/1
  DisbyVy/1 -> Add/2
  DisbyDy/1 -> Product/2, Add1/1, Add/1

== system TosAeb/AEB_Objsel/TA_Processor/PreProcessor/ObjectPrecessor/TAObjInfoCalc/For Each Subsystem/Info_Check1/Basic_Info_check/VxCorrect/Subsystem3/Subsystem2/Subsystem/Subsystem ==
  VbyDx : Inport
  VbyVx : Inport  Port=2
  Add : Sum
  Add1 : Sum  Inputs=+-
  Constant : Constant  Value=2
  Constant1 : Constant  Value=P_TA_ObjSpeedxbyVxandDxFactor
  Divide : Product  Inputs=*/
  Divide1 : Product  Inputs=**
  Relational Operator : RelationalOperator  OutDataTypeStr=boolean  RndMeth=Simplest
  DisLong_flg : Outport
  wires:
  Divide1/1 -> Relational Operator/2
  Constant1/1 -> Divide1/2
  Relational Operator/1 -> DisLong_flg/1
  Add1/1 -> Relational Operator/1
  VbyDx/1 -> Add1/1, Add/1
  Divide/1 -> Add1/2
  Constant/1 -> Divide/2
  Add/1 -> Divide/1
  VbyVx/1 -> Divide1/1, Add/2

== system TosAeb/AEB_Objsel/TA_Processor/PreProcessor/ObjectPrecessor/TAObjInfoCalc/For Each Subsystem/Info_Check1/Basic_Info_check/VxCorrect/Subsystem3/Subsystem2/Subsystem/VbyDx ==
  Fus_ObjDx_Single : Inport
  Add : Sum  Inputs=+-
  Constant : Constant  Value=0.25  OutDataTypeStr=single
  Delay : Delay  DelayLength=5
  Divide : Product  Inputs=*/
  VbyDx : Outport
  wires:
  Constant/1 -> Divide/2
  Divide/1 -> VbyDx/1
  Add/1 -> Divide/1
  Fus_ObjDx_Single/1 -> Delay/1, Add/1
  Delay/1 -> Add/2

== system TosAeb/AEB_Objsel/TA_Processor/PreProcessor/ObjectPrecessor/TAObjInfoCalc/For Each Subsystem/Info_Check1/Basic_Info_check/VxCorrect/Subsystem3/Subsystem2/Subsystem/VbyVx ==
  Fus_ObjVx_Single : Inport
  Constant : Constant  Value=5  OutDataTypeStr=single
  Divide : Product  Inputs=*/
  Sum of Elements : Sum  Inputs=+
  Tapped Delay : simulink/Discrete/Tapped Delay  vinit=0.0  samptime=-1  NumDelays=4  DelayOrder=Oldest  includeCurrent=off
  VbyVx : Outport
  wires:
  Divide/1 -> VbyVx/1
  Tapped Delay/1 -> Sum of Elements/1
  Fus_ObjVx_Single/1 -> Tapped Delay/1
  Constant/1 -> Divide/2
  Sum of Elements/1 -> Divide/1

== system TosAeb/AEB_Objsel/TA_Processor/PreProcessor/ObjectPrecessor/TAObjInfoCalc/For Each Subsystem/Info_Check1/Basic_Info_check/VyCorrect/Subsystem3/Subsystem2/Subsystem/Subsystem ==
  VbyDy : Inport
  VbyVy : Inport  Port=2
  Add : Sum
  Add1 : Sum  Inputs=+-
  Constant : Constant  Value=2
  Constant1 : Constant  Value=P_TA_ObjSpeedybyVyandDyFactor
  Constant2 : Constant  Value=0  OutDataTypeStr=boolean
  Divide : Product  Inputs=*/
  Divide1 : Product  Inputs=**
  Relational Operator : RelationalOperator  OutDataTypeStr=boolean  RndMeth=Simplest
  Terminator : Terminator
  DisLat_flg : Outport
  wires:
  Constant2/1 -> DisLat_flg/1
  VbyVy/1 -> Add/2, Divide1/1
  Add/1 -> Divide/1
  Constant/1 -> Divide/2
  Divide/1 -> Add1/2
  VbyDy/1 -> Add/1, Add1/1
  Add1/1 -> Relational Operator/1
  Relational Operator/1 -> Terminator/1
  Constant1/1 -> Divide1/2
  Divide1/1 -> Relational Operator/2

== system TosAeb/AEB_Objsel/TA_Processor/PreProcessor/ObjectPrecessor/TAObjInfoCalc/For Each Subsystem/Info_Check1/Basic_Info_check/VyCorrect/Subsystem3/Subsystem2/Subsystem/VbyDy ==
  Fus_ObjDy_Single : Inport
  Add : Sum  Inputs=+-
  Constant : Constant  Value=0.1  OutDataTypeStr=single
  Delay : Delay  DelayLength=5
  Divide : Product  Inputs=*/
  VbyDy : Outport
  wires:
  Fus_ObjDy_Single/1 -> Delay/1, Add/1
  Add/1 -> Divide/1
  Divide/1 -> VbyDy/1
  Constant/1 -> Divide/2
  Delay/1 -> Add/2

== system TosAeb/AEB_Objsel/TA_Processor/PreProcessor/ObjectPrecessor/TAObjInfoCalc/For Each Subsystem/Info_Check1/Basic_Info_check/VyCorrect/Subsystem3/Subsystem2/Subsystem/VbyVy ==
  Fus_ObjVy_Single : Inport
  Constant : Constant  Value=5  OutDataTypeStr=single
  Divide : Product  Inputs=*/
  Sum of Elements : Sum  Inputs=+
  Tapped Delay : simulink/Discrete/Tapped Delay  vinit=0.0  samptime=-1  NumDelays=4  DelayOrder=Oldest  includeCurrent=off
  VbyVy : Outport
  wires:
  Constant/1 -> Divide/2
  Fus_ObjVy_Single/1 -> Tapped Delay/1
  Tapped Delay/1 -> Sum of Elements/1
  Divide/1 -> VbyVy/1
  Sum of Elements/1 -> Divide/1

== system TosAeb/FcwAebJa_Step_sys/ALG_AEBSystem/ALG_AEBController/AEB_FuncImpManagementApp/FuncManeuArbCalc/brkFuncManeuArbCalc/axTgtReqValueCalc1/axTgtReqValueCalc4AebControl/aDesiredCalc/If Action Subsystem1/accelAvoidLowLvlBrkCalc/Situation1/Case1 ==
  axAbsObj : Inport
  vx : Inport  Port=2
  dxEgoBrake : Inport  Port=3
  Action Port : ActionPort  ActionPortLabel=if(u1)
  Display : Display
  Divide : Product  Inputs=*/
  Gain : Gain  Gain=2
  Math Function : Math  Operator=square  SignedPower=on  SaturateOnIntegerOverflow=on
  Subtract : Sum  Inputs=+-
  accelAvoid : Outport
  wires:
  Subtract/1 -> Display/1, accelAvoid/1
  axAbsObj/1 -> Subtract/1
  Divide/1 -> Subtract/2
  dxEgoBrake/1 -> Gain/1
  Gain/1 -> Divide/2
  Math Function/1 -> Divide/1
  vx/1 -> Math Function/1
